simulink model "CCMSensor"  (R2022b)
settings: Solver=FixedStepDiscrete  StartTime=0.0  StopTime=10.0  FixedStep=0.01  RelTol=1e-3
undefined symbols (not defined in the file or in its folder): DioChannelType, IcuChannelType, P_DioLevelType
(part 1 of 2)
settings Model: Creator=blue.ge  LastModifiedBy=blue.ge
settings DataIOCC: SaveFormat=StructureWithTime
settings OptimizationCC: DefaultParameterBehavior=Inlined  EnhancedBackFolding=on  PassReuseOutputArgsAs=Individual arguments  ZeroExternalMemoryAtStartup=off  ZeroInternalMemoryAtStartup=off  OptimizeBlockOrder=speed  DifferentSizesBufferReuse=on  OptimizationCustomize=off  EfficientTunableParamExpr=on
settings DebuggingCC: ParameterTunabilityLossMsg=error
settings ModelReferenceCC: ModelReferenceNumInstancesAllowed=Single
settings SFSimCC: MATLABDynamicMemAlloc=off
settings RTWCC: Description=AUTOSAR  SystemTargetFile=autosar.tlc  GenCodeOnly=on  TemplateMakefile=ert_default_tmf
settings CodeAppCC: ForceParamTrailComments=on  IgnoreCustomStorageClasses=off  OperatorAnnotations=on  SimulinkDataObjDesc=on  SFDataObjDesc=on  InsertBlockDesc=on
settings STFCustomTargetCC: TargetLangStandard=C99 (ISO)  MultiwordLength=256  ParMdlRefBuildCompliant=on  CompOptLevelCompliant=on  IncludeMdlTerminateFcn=off  CombineOutputUpdateFcns=on  SuppressErrorStatus=on  ERTCustomFileBanners=on  SupportNonFinite=off  SupportComplex=off  SupportContinuousTime=off  SupportNonInlinedSFcns=off  RemoveResetFunc=on  MATLABClassNameForMDSCustomization=Simulink.SoftwareTarget.ERTCustomization  AutosarCompliant=on  UseToolchainInfoCompliant=on  LookupTableObjectStructAxisOrder=2,1,3,4,...  LUTObjectStructOrderEvenSpacing=Size,Table,Breakpoints  SystemTargetFile=autosar.tlc
settings ERTTargetCC: TargetLangStandard=C99 (ISO)  ConcurrentExecutionCompliant=off  IncludeMdlTerminateFcn=off  SuppressErrorStatus=on  SupportNonFinite=off  SupportComplex=off  ModelStepFunctionPrototypeControlCompliant=off  CPPClassGenCompliant=off  AutosarCompliant=on  LookupTableObjectStructAxisOrder=2,1,3,4,...  LUTObjectStructOrderEvenSpacing=Size,Table,Breakpoints  InlinedParameterPlacement=NonHierarchical  ERTCustomFileTemplate=example_file_process.tlc

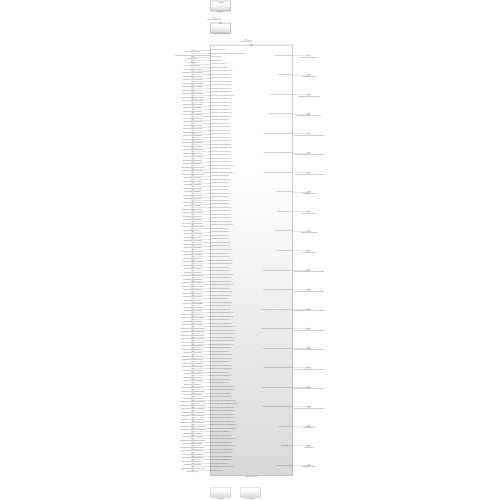
[thumbnail: Simulink's stored preview of the top-level diagram]
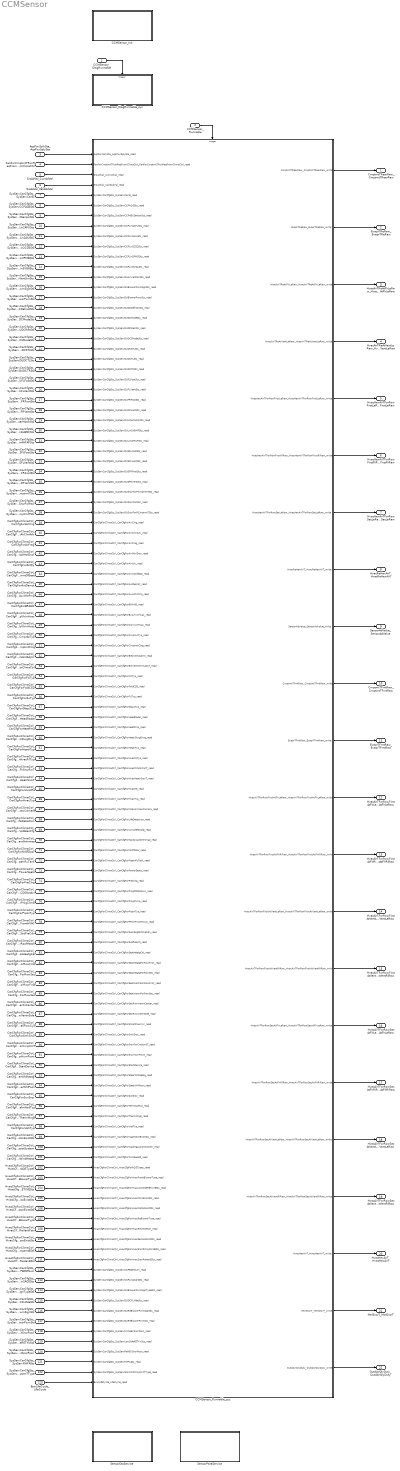
== system CCMSensor ==
  CCMSensor_DiagRunnable : Inport  SampleTime=0.05  OutputFunctionCall=on
  CCMSensor_Runnable : Inport  Port=2  SampleTime=0.02  OutputFunctionCall=on
  AqsPwrSplySta_AqsPwrSplySta : Inport  Port=3  OutDataTypeStr=AqsPwrSplySta  PortDimensions=1
  FanForCmptmtTRunReqFromClimaCtrl_FanForCmptmtTRunReqFromClimaCtrl : Inport  Port=4  OutDataTypeStr=Enum: OnOff1  PortDimensions=1
  SrsAdVal_CurrAdVal : Inport  Port=5  OutDataTypeStr=Bus: SensorAd  PortDimensions=1  BusOutputAsStruct=on
  SrsAdVal_ValidAdVal : Inport  Port=6  OutDataTypeStr=Bus: SensorAd  PortDimensions=1  BusOutputAsStruct=on
  SysServCarCfgSts_SysServCarID : Inport  Port=7  OutDataTypeStr=Enum: SysServCarID  PortDimensions=1
  SysServCarCfgSts_SysServCCPAQSSts : Inport  Port=8  OutDataTypeStr=UInt8  PortDimensions=1
  SysServCarCfgSts_SysServCCPHEXSensorSts : Inport  Port=9  OutDataTypeStr=UInt8  PortDimensions=1
  SysServCarCfgSts_SysServCCPLin2AFUSts : Inport  Port=10  OutDataTypeStr=Boolean  PortDimensions=1
  SysServCarCfgSts_SysServCCPLin2AIUSts : Inport  Port=11  OutDataTypeStr=UInt8  PortDimensions=1
  SysServCarCfgSts_SysServCCPLin2CDSSts : Inport  Port=12  OutDataTypeStr=Boolean  PortDimensions=1
  SysServCarCfgSts_SysServCCPLin2PMSISts : Inport  Port=13  OutDataTypeStr=Boolean  PortDimensions=1
  SysServCarCfgSts_SysServCCPLin3VGASts : Inport  Port=14  OutDataTypeStr=Boolean  PortDimensions=1
  SysServCarCfgSts_SysServDUAirVentSnrSts : Inport  Port=15  OutDataTypeStr=Boolean  PortDimensions=1
  SysServCarCfgSts_SysServDUBlowerPwmSignSts : Inport  Port=16  OutDataTypeStr=Boolean  PortDimensions=1
  SysServCarCfgSts_SysServDUBlowerPwmSts : Inport  Port=17  OutDataTypeStr=Boolean  PortDimensions=1
  SysServCarCfgSts_SysServDUDCDefrostSts : Inport  Port=18  OutDataTypeStr=UInt8  PortDimensions=1
  SysServCarCfgSts_SysServDUDCModeSts : Inport  Port=19  OutDataTypeStr=UInt8  PortDimensions=1
  SysServCarCfgSts_SysServDUDCRecSts : Inport  Port=20  OutDataTypeStr=UInt8  PortDimensions=1
  SysServCarCfgSts_SysServDUDCSModeSts : Inport  Port=21  OutDataTypeStr=UInt8  PortDimensions=1
  SysServCarCfgSts_SysServDUDCSTLSts : Inport  Port=22  OutDataTypeStr=UInt8  PortDimensions=1
  SysServCarCfgSts_SysServDUDCTLSts : Inport  Port=23  OutDataTypeStr=UInt8  PortDimensions=1
  SysServCarCfgSts_SysServDUDCTRSts : Inport  Port=24  OutDataTypeStr=UInt8  PortDimensions=1
  SysServCarCfgSts_SysServDUFLFootSts : Inport  Port=25  OutDataTypeStr=UInt8  PortDimensions=1
  SysServCarCfgSts_SysServDUFLVentSts : Inport  Port=26  OutDataTypeStr=UInt8  PortDimensions=1
  SysServCarCfgSts_SysServDUFRFootSts : Inport  Port=27  OutDataTypeStr=UInt8  PortDimensions=1
  SysServCarCfgSts_SysServDUFRVentSts : Inport  Port=28  OutDataTypeStr=UInt8  PortDimensions=1
  SysServCarCfgSts_SysServDUIncarMotorSts : Inport  Port=29  OutDataTypeStr=Boolean  PortDimensions=1
  SysServCarCfgSts_SysServDULin1HBMFSts : Inport  Port=30  OutDataTypeStr=Boolean  PortDimensions=1
  SysServCarCfgSts_SysServDULin4HFAFSts : Inport  Port=31  OutDataTypeStr=UInt32  PortDimensions=1
  SysServCarCfgSts_SysServDUSFLFootSts : Inport  Port=32  OutDataTypeStr=UInt8  PortDimensions=1
  SysServCarCfgSts_SysServDUSFLVentSts : Inport  Port=33  OutDataTypeStr=UInt8  PortDimensions=1
  SysServCarCfgSts_SysServDUSFRFootSts : Inport  Port=34  OutDataTypeStr=UInt8  PortDimensions=1
  SysServCarCfgSts_SysServDUSFRVentSts : Inport  Port=35  OutDataTypeStr=UInt8  PortDimensions=1
  SysServCarCfgSts_SysServDUSnsrForFCmptmtTSts : Inport  Port=36  OutDataTypeStr=UInt8  PortDimensions=1
  SysServCarCfgSts_SysServDUSnsrForRair : Inport  Port=37  OutDataTypeStr=UInt8  PortDimensions=1
  SysServCarCfgSts_SysServDUSnsrForRCmptmtTSts : Inport  Port=38  OutDataTypeStr=UInt8  PortDimensions=1
  CarCfgForClimaCtrl_CarCfgForAirClng : Inport  Port=39  OutDataTypeStr=Enum: CarCfgForAirClng  PortDimensions=1
  CarCfgForClimaCtrl_CarCfgForAirClnrAdv : Inport  Port=40  OutDataTypeStr=UInt8  PortDimensions=1
  CarCfgForClimaCtrl_CarCfgForAirFrag : Inport  Port=41  OutDataTypeStr=Enum: CarCfgForAirFrag  PortDimensions=1
  CarCfgForClimaCtrl_CarCfgForAirMtrlSnsr : Inport  Port=42  OutDataTypeStr=Enum: CarCfgForAirMtrlSnsrGly  PortDimensions=1
  CarCfgForClimaCtrl_CarCfgForAirQly : Inport  Port=43  OutDataTypeStr=Enum: CarCfgForAirQlyGly  PortDimensions=1
  CarCfgForClimaCtrl_CarCfgForAmntOfSeat : Inport  Port=44  OutDataTypeStr=Enum: CarCfgForAmntOfSeatTyp3  PortDimensions=1
  CarCfgForClimaCtrl_CarCfgForAutDemst : Inport  Port=45  OutDataTypeStr=UInt8  PortDimensions=1
  CarCfgForClimaCtrl_CarCfgForAuxWtrPmp : Inport  Port=46  OutDataTypeStr=Enum: CarCfgForAuxWtrPmp  PortDimensions=1
  CarCfgForClimaCtrl_CarCfgForBRAND : Inport  Port=47  OutDataTypeStr=UInt8  PortDimensions=1
  CarCfgForClimaCtrl_CarCfgForBypVlvInHvac : Inport  Port=48  OutDataTypeStr=Enum: CarCfgForBypVlvInHvac2  PortDimensions=1
  CarCfgForClimaCtrl_CarCfgForClsVlvInHvac : Inport  Port=49  OutDataTypeStr=Enum: CarCfgForClsVlvInHvac  PortDimensions=1
  CarCfgForClimaCtrl_CarCfgForCmprAcTyp : Inport  Port=50  OutDataTypeStr=UInt8  PortDimensions=1
  CarCfgForClimaCtrl_CarCfgForCmptmtClng : Inport  Port=51  OutDataTypeStr=Enum: CarCfgForCmptmtClng  PortDimensions=1
  CarCfgForClimaCtrl_CarCfgForElecVentAdjmt : Inport  Port=52  OutDataTypeStr=Enum: CarCfgForElecVentAdjmt  PortDimensions=1
  CarCfgForClimaCtrl_CarCfgForExtVentnClimaCtrl : Inport  Port=53  OutDataTypeStr=Enum: CarCfgForExtVentnClimaCtrl  PortDimensions=1
  CarCfgForClimaCtrl_CarCfgForFlcTyp : Inport  Port=54  OutDataTypeStr=Enum: CarCfgForFlcTyp  PortDimensions=1
  CarCfgForClimaCtrl_CarCfgForFoldCSD : Inport  Port=55  OutDataTypeStr=Enum: CarCfgForFoldCSD  PortDimensions=1
  CarCfgForClimaCtrl_CarCfgForFuTyp : Inport  Port=56  OutDataTypeStr=Enum: CarCfgForFuTyp  PortDimensions=1
  CarCfgForClimaCtrl_CarCfgForGlassTyp : Inport  Port=57  OutDataTypeStr=Enum: CarCfgForGlassTyp  PortDimensions=1
  CarCfgForClimaCtrl_CarCfgForHeadRadar : Inport  Port=58  OutDataTypeStr=Enum: HeadRadar  PortDimensions=1
  CarCfgForClimaCtrl_CarCfgForHeatPmp : Inport  Port=59  OutDataTypeStr=Enum: CarCfgForHeatPmpTyp  PortDimensions=1
  CarCfgForClimaCtrl_CarCfgForHeatrDurgDrvg : Inport  Port=60  OutDataTypeStr=Enum: CarCfgForHeatrDurgDrvg  PortDimensions=1
  CarCfgForClimaCtrl_CarCfgForHeatrTyp : Inport  Port=61  OutDataTypeStr=UInt8  PortDimensions=1
  CarCfgForClimaCtrl_CarCfgForHvacFilTyp : Inport  Port=62  OutDataTypeStr=Enum: CarCfgForHvacFilTypGly  PortDimensions=1
  CarCfgForClimaCtrl_CarCfgForHvacFlrSnsrForT : Inport  Port=63  OutDataTypeStr=Enum: CarCfgForHvacFlrSnsrForT  PortDimensions=1
  CarCfgForClimaCtrl_CarCfgForHvacHeatrSnsrT : Inport  Port=64  OutDataTypeStr=Enum: CarCfgForHvacHeatrSnsrT  PortDimensions=1
  CarCfgForClimaCtrl_CarCfgForHvacRe : Inport  Port=65  OutDataTypeStr=UInt8  PortDimensions=1
  CarCfgForClimaCtrl_CarCfgForHvacTyp : Inport  Port=66  OutDataTypeStr=UInt8  PortDimensions=1
  CarCfgForClimaCtrl_CarCfgForInteriorViewCamera : Inport  Port=67  OutDataTypeStr=Enum: CarCfgForInteriorViewCamera  PortDimensions=1
  CarCfgForClimaCtrl_CarCfgForLifeDetection : Inport  Port=68  OutDataTypeStr=Enum: LifeDetection  PortDimensions=1
  CarCfgForClimaCtrl_CarCfgForLoVoltElecCfg : Inport  Port=69  OutDataTypeStr=Enum: CarCfgForLoVoltElecCfg  PortDimensions=1
  CarCfgForClimaCtrl_CarCfgForNavSysandIntnmap : Inport  Port=70  OutDataTypeStr=Enum: CarCfgForNavSysandIntnmap  PortDimensions=1
  CarCfgForClimaCtrl_CarCfgForNrOfDoor : Inport  Port=71  OutDataTypeStr=Enum: CarCfgForNrOfDoor2  PortDimensions=1
  CarCfgForClimaCtrl_CarCfgForPipeInFuTank : Inport  Port=72  OutDataTypeStr=Enum: CarCfgForPipeInFuTank  PortDimensions=1
  CarCfgForClimaCtrl_CarCfgForPowerSeats : Inport  Port=73  OutDataTypeStr=Enum: CarCfgForPowerSeats  PortDimensions=1
  CarCfgForClimaCtrl_CarCfgForPreClng : Inport  Port=74  OutDataTypeStr=Enum: CarCfgForPreClng  PortDimensions=1
  CarCfgForClimaCtrl_CarCfgForPrkgCDSNotAvl : Inport  Port=75  OutDataTypeStr=Enum: CarCfgForPrkgCDSNotAvl  PortDimensions=1
  CarCfgForClimaCtrl_CarCfgForPrkgClima : Inport  Port=76  OutDataTypeStr=Enum: CarCfgForPrkgClima  PortDimensions=1
  CarCfgForClimaCtrl_CarCfgForPrpsnTyp : Inport  Port=77  OutDataTypeStr=Enum: CarCfgForPrpsnTypGly  PortDimensions=1
  CarCfgForClimaCtrl_CarCfgForPTCInFrontHVAC : Inport  Port=78  OutDataTypeStr=UInt8  PortDimensions=1
  CarCfgForClimaCtrl_CarCfgForRemEngStrtPreCdn : Inport  Port=79  OutDataTypeStr=Enum: CarCfgForRemEngStrtPreCdn  PortDimensions=1
  CarCfgForClimaCtrl_CarCfgForRoofHatch : Inport  Port=80  OutDataTypeStr=Enum: CarCfgForRoofHatch  PortDimensions=1
  CarCfgForClimaCtrl_CarCfgForSeatHeatgCal : Inport  Port=81  OutDataTypeStr=Enum: CarCfgForSeatHeatgCal1  PortDimensions=1
  CarCfgForClimaCtrl_CarCfgForSeatHeatgForRowFirst : Inport  Port=82  OutDataTypeStr=Enum: CarCfgForSeatHeatgForRowFirst  PortDimensions=1
  CarCfgForClimaCtrl_CarCfgForSeatHeatgForRowSec : Inport  Port=83  OutDataTypeStr=Enum: CarCfgForSeatHeatgForRowSec  PortDimensions=1
  CarCfgForClimaCtrl_CarCfgForSeatVentnForRowFirst : Inport  Port=84  OutDataTypeStr=Enum: CarCfgForSeatVentnForRowFirst1  PortDimensions=1
  CarCfgForClimaCtrl_CarCfgForSeatVentnForRowSec : Inport  Port=85  OutDataTypeStr=Enum: CarCfgForSeatVentnForRowSecGly  PortDimensions=1
  CarCfgForClimaCtrl_CarCfgForSecRowVentnCenter : Inport  Port=86  OutDataTypeStr=Enum: CarCfgForSecRowVentnCenter  PortDimensions=1
  CarCfgForClimaCtrl_CarCfgForSecRowVentnSide : Inport  Port=87  OutDataTypeStr=Enum: CarCfgForSecRowVentnSide  PortDimensions=1
  CarCfgForClimaCtrl_CarCfgForSmallFlowCyc : Inport  Port=88  OutDataTypeStr=Enum: CarCfgForSmallFlowCyc  PortDimensions=1
  CarCfgForClimaCtrl_CarCfgForSmkSnsr : Inport  Port=89  OutDataTypeStr=Enum: CarCfgForSmkSnsr  PortDimensions=1
  CarCfgForClimaCtrl_CarCfgForSnsrForCmptmtT : Inport  Port=90  OutDataTypeStr=Enum: CarCfgForSnsrForCmptmtT  PortDimensions=1
  CarCfgForClimaCtrl_CarCfgForSnsrHumPrsnt : Inport  Port=91  OutDataTypeStr=Enum: CarCfgForSnsrHumPrsnt  PortDimensions=1
  CarCfgForClimaCtrl_CarCfgForStartDevice : Inport  Port=92  OutDataTypeStr=Enum: StartDevice  PortDimensions=1
  CarCfgForClimaCtrl_CarCfgForSteerWhlHeatg : Inport  Port=93  OutDataTypeStr=UInt8  PortDimensions=1
  CarCfgForClimaCtrl_CarCfgForSteerWhlPosn : Inport  Port=94  OutDataTypeStr=Enum: CarCfgForSteerWhlPosn  PortDimensions=1
  CarCfgForClimaCtrl_CarCfgForSunSnsr : Inport  Port=95  OutDataTypeStr=Enum: CarCfgForSunSnsr  PortDimensions=1
  CarCfgForClimaCtrl_CarCfgForTelmNodTyp : Inport  Port=96  OutDataTypeStr=Enum: CarCfgForTelmNodTyp  PortDimensions=1
  CarCfgForClimaCtrl_CarCfgForThermMngt : Inport  Port=97  OutDataTypeStr=Enum: CarCfgForThermMngt  PortDimensions=1
  CarCfgForClimaCtrl_CarCfgForVehTyp : Inport  Port=98  OutDataTypeStr=Enum: CarCfgForVehTyp3  PortDimensions=1
  CarCfgForClimaCtrl_CarCfgForVgaMotorExistSts : Inport  Port=99  OutDataTypeStr=CarCfgForVgaMotorExistSts  PortDimensions=1
  CarCfgForClimaCtrl_CarCfgForWadingAssistSystem : Inport  Port=100  OutDataTypeStr=UInt8  PortDimensions=1
  CarCfgForClimaCtrl_CarCfgForWindHeatd : Inport  Port=101  OutDataTypeStr=Enum: CarCfgForWindHeatd  PortDimensions=1
  HvacCfgForClimaCtrl_HvacCfgForAQSTypes : Inport  Port=102  OutDataTypeStr=UInt8  PortDimensions=1
  HvacCfgForClimaCtrl_HvacCfgForHvacFrontBlowerType : Inport  Port=103  OutDataTypeStr=HvacCfgForHvacFrontBlowerType  PortDimensions=1
  HvacCfgForClimaCtrl_HvacCfgForHvacLowSideRETXVESts : Inport  Port=104  OutDataTypeStr=UInt8  PortDimensions=1
  HvacCfgForClimaCtrl_HvacCfgForHvacMiscExistSts : Inport  Port=105  OutDataTypeStr=HvacCfgForHvacMiscExistSts  PortDimensions=1
  HvacCfgForClimaCtrl_HvacCfgForHvacMotorExistSts : Inport  Port=106  OutDataTypeStr=HvacCfgForHvacMotorExistSts  PortDimensions=1
  HvacCfgForClimaCtrl_HvacCfgForHvacReBlowerType : Inport  Port=107  OutDataTypeStr=UInt8  PortDimensions=1
  HvacCfgForClimaCtrl_HvacCfgForHvacReVentPstn : Inport  Port=108  OutDataTypeStr=UInt8  PortDimensions=1
  HvacCfgForClimaCtrl_HvacCfgForHvacSensorExistSts : Inport  Port=109  OutDataTypeStr=HvacCfgForHvacSensorExistSts  PortDimensions=1
  HvacCfgForClimaCtrl_HvacCfgForHvacSnsrRCmptmtESts : Inport  Port=110  OutDataTypeStr=UInt8  PortDimensions=1
  HvacCfgForClimaCtrl_HvacCfgForHvacSnsrReHexESts : Inport  Port=111  OutDataTypeStr=UInt8  PortDimensions=1
  SysServCarCfgSts_SysServCCPBGMNum : Inport  Port=112  OutDataTypeStr=UInt8  PortDimensions=1
  SysServCarCfgSts_SysServCCPLin2AQSSts : Inport  Port=113  OutDataTypeStr=Boolean  PortDimensions=1
  SysServCarCfgSts_SysServDUBlowerPwmSignTypeSts : Inport  Port=114  OutDataTypeStr=UInt8  PortDimensions=1
  SysServCarCfgSts_SysServDUDCFLMdeSts : Inport  Port=115  OutDataTypeStr=UInt8  PortDimensions=1
  SysServCarCfgSts_SysServDUReBlowerPwmSignSts : Inport  Port=116  OutDataTypeStr=UInt8  PortDimensions=1
  SysServCarCfgSts_SysServDUReBlowerPwmSts : Inport  Port=117  OutDataTypeStr=UInt8  PortDimensions=1
  SysServCarCfgSts_SysServFrntHEXSnsrPosn : Inport  Port=118  OutDataTypeStr=UInt8  PortDimensions=1
  SysServCarCfgSts_SysServLowSideRETXVSts : Inport  Port=119  OutDataTypeStr=UInt8  PortDimensions=1
  SysServCarCfgSts_SysServReHEXSnsrPosn : Inport  Port=120  OutDataTypeStr=UInt8  PortDimensions=1
  SysServCarCfgSts_SysServRHFASts : Inport  Port=121  OutDataTypeStr=UInt8  PortDimensions=1
  SysServCarCfgSts_SysServSnsrForFCmptmtTType : Inport  Port=122  OutDataTypeStr=UInt8  PortDimensions=1
  BswLifeCycle_LifeCycle : Inport  Port=123  OutDataTypeStr=Enum: CCMLifeCycleModeGroup  PortDimensions=1
  CCMSensor_DiagRunnable_sys : Subsystem
  CCMSensor_Init : Subsystem
  CCMSensor_Runnable_sys : Subsystem
  SensorDtcService : Subsystem
  SensorParaService : Subsystem
  CmptmtTRearRaw_CmptmtTRearRaw : Outport  OutDataTypeStr=Bus: CmptmtTFrnt  PortDimensions=1  BusOutputAsStruct=on
  EvaprTReRaw_EvaprTReRaw : Outport  Port=2  OutDataTypeStr=Bus: EvaprTFrnt  PortDimensions=1  BusOutputAsStruct=on
  HvacAirTReAtFlrLeRaw_HvacAirTReAtFlrLeRaw : Outport  Port=3  OutDataTypeStr=Bus: HvacAirTReAtFlrLe  PortDimensions=1  BusOutputAsStruct=on
  HvacAirTReAtVentLeRaw_HvacAirTReAtVentLeRaw : Outport  Port=4  OutDataTypeStr=Bus: HvacAirTReAtVentLe  PortDimensions=1  BusOutputAsStruct=on
  HvacHexAirTForRowFirstLeRaw_HvacHexAirTForRowFirstLeRaw : Outport  Port=5  OutDataTypeStr=Bus: HvacHexAirTForRowFirstLe  PortDimensions=1  BusOutputAsStruct=on
  HvacHexAirTForRowFirstRiRaw_HvacHexAirTForRowFirstRiRaw : Outport  Port=6  OutDataTypeStr=Bus: HvacHexAirTForRowFirstRi  PortDimensions=1  BusOutputAsStruct=on
  HvacHexAirTForRowSecLeRaw_HvacHexAirTForRowSecLeRaw : Outport  Port=7  OutDataTypeStr=Bus: HvacHexAirTForRowSecLe  PortDimensions=1  BusOutputAsStruct=on
  HvacReHexAirT_HvacReHexAirT : Outport  Port=8  OutDataTypeStr=Bus: HvacAirTForHeatrFrnt  PortDimensions=1  BusOutputAsStruct=on
  SensorAdValue_SensorAdValue : Outport  Port=9  OutDataTypeStr=Bus: SensorAd  PortDimensions=1  BusOutputAsStruct=on
  CmptmtTFrntRaw_CmptmtTFrntRaw : Outport  Port=10  OutDataTypeStr=Bus: CmptmtTFrnt  PortDimensions=1  BusOutputAsStruct=on
  EvaprTFrntRaw_EvaprTFrntRaw : Outport  Port=11  OutDataTypeStr=Bus: EvaprTFrnt  PortDimensions=1  BusOutputAsStruct=on
  HvacAirTForRowFirstAtFlrLeRaw_HvacAirTForRowFirstAtFlrLeRaw : Outport  Port=12  OutDataTypeStr=Bus: HvacAirTForRowFirstAtFlrLe  PortDimensions=1  BusOutputAsStruct=on
  HvacAirTForRowFirstAtFlrRiRaw_HvacAirTForRowFirstAtFlrRiRaw : Outport  Port=13  OutDataTypeStr=Bus: HvacAirTForRowFirstAtFlrRi  PortDimensions=1  BusOutputAsStruct=on
  HvacAirTForRowFirstAtVentLeRaw_HvacAirTForRowFirstAtVentLeRaw : Outport  Port=14  OutDataTypeStr=Bus: HvacAirTForRowFirstAtVentLe  PortDimensions=1  BusOutputAsStruct=on
  HvacAirTForRowFirstAtVentRiRaw_HvacAirTForRowFirstAtVentRiRaw : Outport  Port=15  OutDataTypeStr=Bus: HvacAirTForRowFirstAtVentRi  PortDimensions=1  BusOutputAsStruct=on
  HvacAirTForRowSecAtFlrLeRaw_HvacAirTForRowSecAtFlrLeRaw : Outport  Port=16  OutDataTypeStr=Bus: HvacAirTForRowSecAtFlrLe  PortDimensions=1  BusOutputAsStruct=on
  HvacAirTForRowSecAtFlrRiRaw_HvacAirTForRowSecAtFlrRiRaw : Outport  Port=17  OutDataTypeStr=Bus: HvacAirTForRowSecAtFlrRi  PortDimensions=1  BusOutputAsStruct=on
  HvacAirTForRowSecAtVentLeRaw_HvacAirTForRowSecAtVentLeRaw : Outport  Port=18  OutDataTypeStr=Bus: HvacAirTForRowSecAtVentLe  PortDimensions=1  BusOutputAsStruct=on
  HvacAirTForRowSecAtVentRiRaw_HvacAirTForRowSecAtVentRiRaw : Outport  Port=19  OutDataTypeStr=Bus: HvacAirTForRowSecAtVentRi  PortDimensions=1  BusOutputAsStruct=on
  HvacHexAirT_HvacHexAirT : Outport  Port=20  OutDataTypeStr=Bus: HvacAirTForHeatrFrnt  PortDimensions=1  BusOutputAsStruct=on
  MtrlSnsrT_MtrlSnsrT : Outport  Port=21  OutDataTypeStr=Bus: MtrlSnsrT  PortDimensions=1  BusOutputAsStruct=on
  OutdAirQlyDuty_OutdAirQlyDuty : Outport  Port=22  OutDataTypeStr=Bus: OutdAirQlyDutyWithQly  PortDimensions=1  BusOutputAsStruct=on
  wires:
  AqsPwrSplySta_AqsPwrSplySta/1 -> CCMSensor_Runnable_sys/1(AqsPwrSplySta_AqsPwrSplySta_read)
  FanForCmptmtTRunReqFromClimaCtrl_FanForCmptmtTRunReqFromClimaCtrl/1 -> CCMSensor_Runnable_sys/2(FanForCmptmtTRunReqFromClimaCtrl_FanForCmptmtTRunReqFromClimaCtrl_read)
  SrsAdVal_CurrAdVal/1 -> CCMSensor_Runnable_sys/3(SrsAdVal_CurrAdVal_read)
  SrsAdVal_ValidAdVal/1 -> CCMSensor_Runnable_sys/4(SrsAdVal_ValidAdVal_read)
  SysServCarCfgSts_SysServCarID/1 -> CCMSensor_Runnable_sys/5(SysServCarCfgSts_SysServCarID_read)
  SysServCarCfgSts_SysServCCPAQSSts/1 -> CCMSensor_Runnable_sys/6(SysServCarCfgSts_SysServCCPAQSSts_read)
  SysServCarCfgSts_SysServCCPHEXSensorSts/1 -> CCMSensor_Runnable_sys/7(SysServCarCfgSts_SysServCCPHEXSensorSts_read)
  SysServCarCfgSts_SysServCCPLin2AFUSts/1 -> CCMSensor_Runnable_sys/8(SysServCarCfgSts_SysServCCPLin2AFUSts_read)
  SysServCarCfgSts_SysServCCPLin2AIUSts/1 -> CCMSensor_Runnable_sys/9(SysServCarCfgSts_SysServCCPLin2AIUSts_read)
  SysServCarCfgSts_SysServCCPLin2CDSSts/1 -> CCMSensor_Runnable_sys/10(SysServCarCfgSts_SysServCCPLin2CDSSts_read)
  SysServCarCfgSts_SysServCCPLin2PMSISts/1 -> CCMSensor_Runnable_sys/11(SysServCarCfgSts_SysServCCPLin2PMSISts_read)
  SysServCarCfgSts_SysServCCPLin3VGASts/1 -> CCMSensor_Runnable_sys/12(SysServCarCfgSts_SysServCCPLin3VGASts_read)
  SysServCarCfgSts_SysServDUAirVentSnrSts/1 -> CCMSensor_Runnable_sys/13(SysServCarCfgSts_SysServDUAirVentSnrSts_read)
  SysServCarCfgSts_SysServDUBlowerPwmSignSts/1 -> CCMSensor_Runnable_sys/14(SysServCarCfgSts_SysServDUBlowerPwmSignSts_read)
  SysServCarCfgSts_SysServDUBlowerPwmSts/1 -> CCMSensor_Runnable_sys/15(SysServCarCfgSts_SysServDUBlowerPwmSts_read)
  SysServCarCfgSts_SysServDUDCDefrostSts/1 -> CCMSensor_Runnable_sys/16(SysServCarCfgSts_SysServDUDCDefrostSts_read)
  SysServCarCfgSts_SysServDUDCModeSts/1 -> CCMSensor_Runnable_sys/17(SysServCarCfgSts_SysServDUDCModeSts_read)
  SysServCarCfgSts_SysServDUDCRecSts/1 -> CCMSensor_Runnable_sys/18(SysServCarCfgSts_SysServDUDCRecSts_read)
  SysServCarCfgSts_SysServDUDCSModeSts/1 -> CCMSensor_Runnable_sys/19(SysServCarCfgSts_SysServDUDCSModeSts_read)
  SysServCarCfgSts_SysServDUDCSTLSts/1 -> CCMSensor_Runnable_sys/20(SysServCarCfgSts_SysServDUDCSTLSts_read)
  SysServCarCfgSts_SysServDUDCTLSts/1 -> CCMSensor_Runnable_sys/21(SysServCarCfgSts_SysServDUDCTLSts_read)
  SysServCarCfgSts_SysServDUDCTRSts/1 -> CCMSensor_Runnable_sys/22(SysServCarCfgSts_SysServDUDCTRSts_read)
  SysServCarCfgSts_SysServDUFLFootSts/1 -> CCMSensor_Runnable_sys/23(SysServCarCfgSts_SysServDUFLFootSts_read)
  SysServCarCfgSts_SysServDUFLVentSts/1 -> CCMSensor_Runnable_sys/24(SysServCarCfgSts_SysServDUFLVentSts_read)
  SysServCarCfgSts_SysServDUFRFootSts/1 -> CCMSensor_Runnable_sys/25(SysServCarCfgSts_SysServDUFRFootSts_read)
  SysServCarCfgSts_SysServDUFRVentSts/1 -> CCMSensor_Runnable_sys/26(SysServCarCfgSts_SysServDUFRVentSts_read)
  SysServCarCfgSts_SysServDUIncarMotorSts/1 -> CCMSensor_Runnable_sys/27(SysServCarCfgSts_SysServDUIncarMotorSts_read)
  SysServCarCfgSts_SysServDULin1HBMFSts/1 -> CCMSensor_Runnable_sys/28(SysServCarCfgSts_SysServDULin1HBMFSts_read)
  SysServCarCfgSts_SysServDULin4HFAFSts/1 -> CCMSensor_Runnable_sys/29(SysServCarCfgSts_SysServDULin4HFAFSts_read)
  SysServCarCfgSts_SysServDUSFLFootSts/1 -> CCMSensor_Runnable_sys/30(SysServCarCfgSts_SysServDUSFLFootSts_read)
  SysServCarCfgSts_SysServDUSFLVentSts/1 -> CCMSensor_Runnable_sys/31(SysServCarCfgSts_SysServDUSFLVentSts_read)
  SysServCarCfgSts_SysServDUSFRFootSts/1 -> CCMSensor_Runnable_sys/32(SysServCarCfgSts_SysServDUSFRFootSts_read)
  SysServCarCfgSts_SysServDUSFRVentSts/1 -> CCMSensor_Runnable_sys/33(SysServCarCfgSts_SysServDUSFRVentSts_read)
  SysServCarCfgSts_SysServDUSnsrForFCmptmtTSts/1 -> CCMSensor_Runnable_sys/34(SysServCarCfgSts_SysServDUSnsrForFCmptmtTSts_read)
  SysServCarCfgSts_SysServDUSnsrForRair/1 -> CCMSensor_Runnable_sys/35(SysServCarCfgSts_SysServDUSnsrForRair_read)
  SysServCarCfgSts_SysServDUSnsrForRCmptmtTSts/1 -> CCMSensor_Runnable_sys/36(SysServCarCfgSts_SysServDUSnsrForRCmptmtTSts_read)
  CarCfgForClimaCtrl_CarCfgForAirClng/1 -> CCMSensor_Runnable_sys/37(CarCfgForClimaCtrl_CarCfgForAirClng_read)
  CarCfgForClimaCtrl_CarCfgForAirClnrAdv/1 -> CCMSensor_Runnable_sys/38(CarCfgForClimaCtrl_CarCfgForAirClnrAdv_read)
  CarCfgForClimaCtrl_CarCfgForAirFrag/1 -> CCMSensor_Runnable_sys/39(CarCfgForClimaCtrl_CarCfgForAirFrag_read)
  CarCfgForClimaCtrl_CarCfgForAirMtrlSnsr/1 -> CCMSensor_Runnable_sys/40(CarCfgForClimaCtrl_CarCfgForAirMtrlSnsr_read)
  CarCfgForClimaCtrl_CarCfgForAirQly/1 -> CCMSensor_Runnable_sys/41(CarCfgForClimaCtrl_CarCfgForAirQly_read)
  CarCfgForClimaCtrl_CarCfgForAmntOfSeat/1 -> CCMSensor_Runnable_sys/42(CarCfgForClimaCtrl_CarCfgForAmntOfSeat_read)
  CarCfgForClimaCtrl_CarCfgForAutDemst/1 -> CCMSensor_Runnable_sys/43(CarCfgForClimaCtrl_CarCfgForAutDemst_read)
  CarCfgForClimaCtrl_CarCfgForAuxWtrPmp/1 -> CCMSensor_Runnable_sys/44(CarCfgForClimaCtrl_CarCfgForAuxWtrPmp_read)
  CarCfgForClimaCtrl_CarCfgForBRAND/1 -> CCMSensor_Runnable_sys/45(CarCfgForClimaCtrl_CarCfgForBRAND_read)
  CarCfgForClimaCtrl_CarCfgForBypVlvInHvac/1 -> CCMSensor_Runnable_sys/46(CarCfgForClimaCtrl_CarCfgForBypVlvInHvac_read)
  CarCfgForClimaCtrl_CarCfgForClsVlvInHvac/1 -> CCMSensor_Runnable_sys/47(CarCfgForClimaCtrl_CarCfgForClsVlvInHvac_read)
  CarCfgForClimaCtrl_CarCfgForCmprAcTyp/1 -> CCMSensor_Runnable_sys/48(CarCfgForClimaCtrl_CarCfgForCmprAcTyp_read)
  CarCfgForClimaCtrl_CarCfgForCmptmtClng/1 -> CCMSensor_Runnable_sys/49(CarCfgForClimaCtrl_CarCfgForCmptmtClng_read)
  CarCfgForClimaCtrl_CarCfgForElecVentAdjmt/1 -> CCMSensor_Runnable_sys/50(CarCfgForClimaCtrl_CarCfgForElecVentAdjmt_read)
  CarCfgForClimaCtrl_CarCfgForExtVentnClimaCtrl/1 -> CCMSensor_Runnable_sys/51(CarCfgForClimaCtrl_CarCfgForExtVentnClimaCtrl_read)
  CarCfgForClimaCtrl_CarCfgForFlcTyp/1 -> CCMSensor_Runnable_sys/52(CarCfgForClimaCtrl_CarCfgForFlcTyp_read)
  CarCfgForClimaCtrl_CarCfgForFoldCSD/1 -> CCMSensor_Runnable_sys/53(CarCfgForClimaCtrl_CarCfgForFoldCSD_read)
  CarCfgForClimaCtrl_CarCfgForFuTyp/1 -> CCMSensor_Runnable_sys/54(CarCfgForClimaCtrl_CarCfgForFuTyp_read)
  CarCfgForClimaCtrl_CarCfgForGlassTyp/1 -> CCMSensor_Runnable_sys/55(CarCfgForClimaCtrl_CarCfgForGlassTyp_read)
  CarCfgForClimaCtrl_CarCfgForHeadRadar/1 -> CCMSensor_Runnable_sys/56(CarCfgForClimaCtrl_CarCfgForHeadRadar_read)
  CarCfgForClimaCtrl_CarCfgForHeatPmp/1 -> CCMSensor_Runnable_sys/57(CarCfgForClimaCtrl_CarCfgForHeatPmp_read)
  CarCfgForClimaCtrl_CarCfgForHeatrDurgDrvg/1 -> CCMSensor_Runnable_sys/58(CarCfgForClimaCtrl_CarCfgForHeatrDurgDrvg_read)
  CarCfgForClimaCtrl_CarCfgForHeatrTyp/1 -> CCMSensor_Runnable_sys/59(CarCfgForClimaCtrl_CarCfgForHeatrTyp_read)
  CarCfgForClimaCtrl_CarCfgForHvacFilTyp/1 -> CCMSensor_Runnable_sys/60(CarCfgForClimaCtrl_CarCfgForHvacFilTyp_read)
  CarCfgForClimaCtrl_CarCfgForHvacFlrSnsrForT/1 -> CCMSensor_Runnable_sys/61(CarCfgForClimaCtrl_CarCfgForHvacFlrSnsrForT_read)
  CarCfgForClimaCtrl_CarCfgForHvacHeatrSnsrT/1 -> CCMSensor_Runnable_sys/62(CarCfgForClimaCtrl_CarCfgForHvacHeatrSnsrT_read)
  CarCfgForClimaCtrl_CarCfgForHvacRe/1 -> CCMSensor_Runnable_sys/63(CarCfgForClimaCtrl_CarCfgForHvacRe_read)
  CarCfgForClimaCtrl_CarCfgForHvacTyp/1 -> CCMSensor_Runnable_sys/64(CarCfgForClimaCtrl_CarCfgForHvacTyp_read)
  CarCfgForClimaCtrl_CarCfgForInteriorViewCamera/1 -> CCMSensor_Runnable_sys/65(CarCfgForClimaCtrl_CarCfgForInteriorViewCamera_read)
  CarCfgForClimaCtrl_CarCfgForLifeDetection/1 -> CCMSensor_Runnable_sys/66(CarCfgForClimaCtrl_CarCfgForLifeDetection_read)
  CarCfgForClimaCtrl_CarCfgForLoVoltElecCfg/1 -> CCMSensor_Runnable_sys/67(CarCfgForClimaCtrl_CarCfgForLoVoltElecCfg_read)
  CarCfgForClimaCtrl_CarCfgForNavSysandIntnmap/1 -> CCMSensor_Runnable_sys/68(CarCfgForClimaCtrl_CarCfgForNavSysandIntnmap_read)
  CarCfgForClimaCtrl_CarCfgForNrOfDoor/1 -> CCMSensor_Runnable_sys/69(CarCfgForClimaCtrl_CarCfgForNrOfDoor_read)
  CarCfgForClimaCtrl_CarCfgForPipeInFuTank/1 -> CCMSensor_Runnable_sys/70(CarCfgForClimaCtrl_CarCfgForPipeInFuTank_read)
  CarCfgForClimaCtrl_CarCfgForPowerSeats/1 -> CCMSensor_Runnable_sys/71(CarCfgForClimaCtrl_CarCfgForPowerSeats_read)
  CarCfgForClimaCtrl_CarCfgForPreClng/1 -> CCMSensor_Runnable_sys/72(CarCfgForClimaCtrl_CarCfgForPreClng_read)
  CarCfgForClimaCtrl_CarCfgForPrkgCDSNotAvl/1 -> CCMSensor_Runnable_sys/73(CarCfgForClimaCtrl_CarCfgForPrkgCDSNotAvl_read)
  CarCfgForClimaCtrl_CarCfgForPrkgClima/1 -> CCMSensor_Runnable_sys/74(CarCfgForClimaCtrl_CarCfgForPrkgClima_read)
  CarCfgForClimaCtrl_CarCfgForPrpsnTyp/1 -> CCMSensor_Runnable_sys/75(CarCfgForClimaCtrl_CarCfgForPrpsnTyp_read)
  CarCfgForClimaCtrl_CarCfgForPTCInFrontHVAC/1 -> CCMSensor_Runnable_sys/76(CarCfgForClimaCtrl_CarCfgForPTCInFrontHVAC_read)
  CarCfgForClimaCtrl_CarCfgForRemEngStrtPreCdn/1 -> CCMSensor_Runnable_sys/77(CarCfgForClimaCtrl_CarCfgForRemEngStrtPreCdn_read)
  CarCfgForClimaCtrl_CarCfgForRoofHatch/1 -> CCMSensor_Runnable_sys/78(CarCfgForClimaCtrl_CarCfgForRoofHatch_read)
  CarCfgForClimaCtrl_CarCfgForSeatHeatgCal/1 -> CCMSensor_Runnable_sys/79(CarCfgForClimaCtrl_CarCfgForSeatHeatgCal_read)
  CarCfgForClimaCtrl_CarCfgForSeatHeatgForRowFirst/1 -> CCMSensor_Runnable_sys/80(CarCfgForClimaCtrl_CarCfgForSeatHeatgForRowFirst_read)
  CarCfgForClimaCtrl_CarCfgForSeatHeatgForRowSec/1 -> CCMSensor_Runnable_sys/81(CarCfgForClimaCtrl_CarCfgForSeatHeatgForRowSec_read)
  CarCfgForClimaCtrl_CarCfgForSeatVentnForRowFirst/1 -> CCMSensor_Runnable_sys/82(CarCfgForClimaCtrl_CarCfgForSeatVentnForRowFirst_read)
  CarCfgForClimaCtrl_CarCfgForSeatVentnForRowSec/1 -> CCMSensor_Runnable_sys/83(CarCfgForClimaCtrl_CarCfgForSeatVentnForRowSec_read)
  CarCfgForClimaCtrl_CarCfgForSecRowVentnCenter/1 -> CCMSensor_Runnable_sys/84(CarCfgForClimaCtrl_CarCfgForSecRowVentnCenter_read)
  CarCfgForClimaCtrl_CarCfgForSecRowVentnSide/1 -> CCMSensor_Runnable_sys/85(CarCfgForClimaCtrl_CarCfgForSecRowVentnSide_read)
  CarCfgForClimaCtrl_CarCfgForSmallFlowCyc/1 -> CCMSensor_Runnable_sys/86(CarCfgForClimaCtrl_CarCfgForSmallFlowCyc_read)
  CarCfgForClimaCtrl_CarCfgForSmkSnsr/1 -> CCMSensor_Runnable_sys/87(CarCfgForClimaCtrl_CarCfgForSmkSnsr_read)
  CarCfgForClimaCtrl_CarCfgForSnsrForCmptmtT/1 -> CCMSensor_Runnable_sys/88(CarCfgForClimaCtrl_CarCfgForSnsrForCmptmtT_read)
  CarCfgForClimaCtrl_CarCfgForSnsrHumPrsnt/1 -> CCMSensor_Runnable_sys/89(CarCfgForClimaCtrl_CarCfgForSnsrHumPrsnt_read)
  CarCfgForClimaCtrl_CarCfgForStartDevice/1 -> CCMSensor_Runnable_sys/90(CarCfgForClimaCtrl_CarCfgForStartDevice_read)
  CarCfgForClimaCtrl_CarCfgForSteerWhlHeatg/1 -> CCMSensor_Runnable_sys/91(CarCfgForClimaCtrl_CarCfgForSteerWhlHeatg_read)
  CarCfgForClimaCtrl_CarCfgForSteerWhlPosn/1 -> CCMSensor_Runnable_sys/92(CarCfgForClimaCtrl_CarCfgForSteerWhlPosn_read)
  CarCfgForClimaCtrl_CarCfgForSunSnsr/1 -> CCMSensor_Runnable_sys/93(CarCfgForClimaCtrl_CarCfgForSunSnsr_read)
  CarCfgForClimaCtrl_CarCfgForTelmNodTyp/1 -> CCMSensor_Runnable_sys/94(CarCfgForClimaCtrl_CarCfgForTelmNodTyp_read)
  CarCfgForClimaCtrl_CarCfgForThermMngt/1 -> CCMSensor_Runnable_sys/95(CarCfgForClimaCtrl_CarCfgForThermMngt_read)
  CarCfgForClimaCtrl_CarCfgForVehTyp/1 -> CCMSensor_Runnable_sys/96(CarCfgForClimaCtrl_CarCfgForVehTyp_read)
  CarCfgForClimaCtrl_CarCfgForVgaMotorExistSts/1 -> CCMSensor_Runnable_sys/97(CarCfgForClimaCtrl_CarCfgForVgaMotorExistSts_read)
  CarCfgForClimaCtrl_CarCfgForWadingAssistSystem/1 -> CCMSensor_Runnable_sys/98(CarCfgForClimaCtrl_CarCfgForWadingAssistSystem_read)
  CarCfgForClimaCtrl_CarCfgForWindHeatd/1 -> CCMSensor_Runnable_sys/99(CarCfgForClimaCtrl_CarCfgForWindHeatd_read)
  HvacCfgForClimaCtrl_HvacCfgForAQSTypes/1 -> CCMSensor_Runnable_sys/100(HvacCfgForClimaCtrl_HvacCfgForAQSTypes_read)
  HvacCfgForClimaCtrl_HvacCfgForHvacFrontBlowerType/1 -> CCMSensor_Runnable_sys/101(HvacCfgForClimaCtrl_HvacCfgForHvacFrontBlowerType_read)
  HvacCfgForClimaCtrl_HvacCfgForHvacLowSideRETXVESts/1 -> CCMSensor_Runnable_sys/102(HvacCfgForClimaCtrl_HvacCfgForHvacLowSideRETXVESts_read)
  HvacCfgForClimaCtrl_HvacCfgForHvacMiscExistSts/1 -> CCMSensor_Runnable_sys/103(HvacCfgForClimaCtrl_HvacCfgForHvacMiscExistSts_read)
  HvacCfgForClimaCtrl_HvacCfgForHvacMotorExistSts/1 -> CCMSensor_Runnable_sys/104(HvacCfgForClimaCtrl_HvacCfgForHvacMotorExistSts_read)
  HvacCfgForClimaCtrl_HvacCfgForHvacReBlowerType/1 -> CCMSensor_Runnable_sys/105(HvacCfgForClimaCtrl_HvacCfgForHvacReBlowerType_read)
  HvacCfgForClimaCtrl_HvacCfgForHvacReVentPstn/1 -> CCMSensor_Runnable_sys/106(HvacCfgForClimaCtrl_HvacCfgForHvacReVentPstn_read)
  HvacCfgForClimaCtrl_HvacCfgForHvacSensorExistSts/1 -> CCMSensor_Runnable_sys/107(HvacCfgForClimaCtrl_HvacCfgForHvacSensorExistSts_read)
  HvacCfgForClimaCtrl_HvacCfgForHvacSnsrRCmptmtESts/1 -> CCMSensor_Runnable_sys/108(HvacCfgForClimaCtrl_HvacCfgForHvacSnsrRCmptmtESts_read)
  HvacCfgForClimaCtrl_HvacCfgForHvacSnsrReHexESts/1 -> CCMSensor_Runnable_sys/109(HvacCfgForClimaCtrl_HvacCfgForHvacSnsrReHexESts_read)
  SysServCarCfgSts_SysServCCPBGMNum/1 -> CCMSensor_Runnable_sys/110(SysServCarCfgSts_SysServCCPBGMNum_read)
  SysServCarCfgSts_SysServCCPLin2AQSSts/1 -> CCMSensor_Runnable_sys/111(SysServCarCfgSts_SysServCCPLin2AQSSts_read)
  SysServCarCfgSts_SysServDUBlowerPwmSignTypeSts/1 -> CCMSensor_Runnable_sys/112(SysServCarCfgSts_SysServDUBlowerPwmSignTypeSts_read)
  SysServCarCfgSts_SysServDUDCFLMdeSts/1 -> CCMSensor_Runnable_sys/113(SysServCarCfgSts_SysServDUDCFLMdeSts_read)
  SysServCarCfgSts_SysServDUReBlowerPwmSignSts/1 -> CCMSensor_Runnable_sys/114(SysServCarCfgSts_SysServDUReBlowerPwmSignSts_read)
  SysServCarCfgSts_SysServDUReBlowerPwmSts/1 -> CCMSensor_Runnable_sys/115(SysServCarCfgSts_SysServDUReBlowerPwmSts_read)
  SysServCarCfgSts_SysServFrntHEXSnsrPosn/1 -> CCMSensor_Runnable_sys/116(SysServCarCfgSts_SysServFrntHEXSnsrPosn_read)
  SysServCarCfgSts_SysServLowSideRETXVSts/1 -> CCMSensor_Runnable_sys/117(SysServCarCfgSts_SysServLowSideRETXVSts_read)
  SysServCarCfgSts_SysServReHEXSnsrPosn/1 -> CCMSensor_Runnable_sys/118(SysServCarCfgSts_SysServReHEXSnsrPosn_read)
  SysServCarCfgSts_SysServRHFASts/1 -> CCMSensor_Runnable_sys/119(SysServCarCfgSts_SysServRHFASts_read)
  SysServCarCfgSts_SysServSnsrForFCmptmtTType/1 -> CCMSensor_Runnable_sys/120(SysServCarCfgSts_SysServSnsrForFCmptmtTType_read)
  CCMSensor_Runnable_sys/1(CmptmtTRearRaw_CmptmtTRearRaw_write) -> CmptmtTRearRaw_CmptmtTRearRaw/1
  CCMSensor_Runnable_sys/2(EvaprTReRaw_EvaprTReRaw_write) -> EvaprTReRaw_EvaprTReRaw/1
  CCMSensor_Runnable_sys/3(HvacAirTReAtFlrLeRaw_HvacAirTReAtFlrLeRaw_write) -> HvacAirTReAtFlrLeRaw_HvacAirTReAtFlrLeRaw/1
  CCMSensor_Runnable_sys/4(HvacAirTReAtVentLeRaw_HvacAirTReAtVentLeRaw_write) -> HvacAirTReAtVentLeRaw_HvacAirTReAtVentLeRaw/1
  CCMSensor_Runnable_sys/5(HvacHexAirTForRowFirstLeRaw_HvacHexAirTForRowFirstLeRaw_write) -> HvacHexAirTForRowFirstLeRaw_HvacHexAirTForRowFirstLeRaw/1
  CCMSensor_Runnable_sys/6(HvacHexAirTForRowFirstRiRaw_HvacHexAirTForRowFirstRiRaw_write) -> HvacHexAirTForRowFirstRiRaw_HvacHexAirTForRowFirstRiRaw/1
  CCMSensor_Runnable_sys/7(HvacHexAirTForRowSecLeRaw_HvacHexAirTForRowSecLeRaw_write) -> HvacHexAirTForRowSecLeRaw_HvacHexAirTForRowSecLeRaw/1
  CCMSensor_Runnable_sys/8(HvacReHexAirT_HvacReHexAirT_write) -> HvacReHexAirT_HvacReHexAirT/1
  CCMSensor_Runnable_sys/9(SensorAdValue_SensorAdValue_write) -> SensorAdValue_SensorAdValue/1
  CCMSensor_Runnable_sys/10(CmptmtTFrntRaw_CmptmtTFrntRaw_write) -> CmptmtTFrntRaw_CmptmtTFrntRaw/1
  CCMSensor_Runnable_sys/11(EvaprTFrntRaw_EvaprTFrntRaw_write) -> EvaprTFrntRaw_EvaprTFrntRaw/1
  CCMSensor_Runnable_sys/12(HvacAirTForRowFirstAtFlrLeRaw_HvacAirTForRowFirstAtFlrLeRaw_write) -> HvacAirTForRowFirstAtFlrLeRaw_HvacAirTForRowFirstAtFlrLeRaw/1
  CCMSensor_Runnable_sys/13(HvacAirTForRowFirstAtFlrRiRaw_HvacAirTForRowFirstAtFlrRiRaw_write) -> HvacAirTForRowFirstAtFlrRiRaw_HvacAirTForRowFirstAtFlrRiRaw/1
  CCMSensor_Runnable_sys/14(HvacAirTForRowFirstAtVentLeRaw_HvacAirTForRowFirstAtVentLeRaw_write) -> HvacAirTForRowFirstAtVentLeRaw_HvacAirTForRowFirstAtVentLeRaw/1
  CCMSensor_Runnable_sys/15(HvacAirTForRowFirstAtVentRiRaw_HvacAirTForRowFirstAtVentRiRaw_write) -> HvacAirTForRowFirstAtVentRiRaw_HvacAirTForRowFirstAtVentRiRaw/1
  CCMSensor_Runnable_sys/16(HvacAirTForRowSecAtFlrLeRaw_HvacAirTForRowSecAtFlrLeRaw_write) -> HvacAirTForRowSecAtFlrLeRaw_HvacAirTForRowSecAtFlrLeRaw/1
  CCMSensor_Runnable_sys/17(HvacAirTForRowSecAtFlrRiRaw_HvacAirTForRowSecAtFlrRiRaw_write) -> HvacAirTForRowSecAtFlrRiRaw_HvacAirTForRowSecAtFlrRiRaw/1
  CCMSensor_Runnable_sys/18(HvacAirTForRowSecAtVentLeRaw_HvacAirTForRowSecAtVentLeRaw_write) -> HvacAirTForRowSecAtVentLeRaw_HvacAirTForRowSecAtVentLeRaw/1
  CCMSensor_Runnable_sys/19(HvacAirTForRowSecAtVentRiRaw_HvacAirTForRowSecAtVentRiRaw_write) -> HvacAirTForRowSecAtVentRiRaw_HvacAirTForRowSecAtVentRiRaw/1
  CCMSensor_Runnable_sys/20(HvacHexAirT_HvacHexAirT_write) -> HvacHexAirT_HvacHexAirT/1
  CCMSensor_Runnable_sys/21(MtrlSnsrT_MtrlSnsrT_write) -> MtrlSnsrT_MtrlSnsrT/1
  CCMSensor_Runnable_sys/22(OutdAirQlyDuty_OutdAirQlyDuty_write) -> OutdAirQlyDuty_OutdAirQlyDuty/1
  BswLifeCycle_LifeCycle/1 -> CCMSensor_Runnable_sys/121(BswLifeCycle_LifeCycle_read)
  CCMSensor_DiagRunnable/1 -> CCMSensor_DiagRunnable_sys/Trigger
  CCMSensor_Runnable/1 -> CCMSensor_Runnable_sys/Trigger

== system CCMSensor/CCMSensor_DiagRunnable_sys ==
  function : TriggerPort  TriggerType=function-call  VariantControl=(inherit)  SampleTimeType=periodic  SampleTime=-1

== system CCMSensor/CCMSensor_Init ==
  Event Listener : EventListener  EventName=reset
